simulink model "MCVT_Integration"  (R2020a)
settings: Solver=ode8  StartTime=0.0  StopTime=T_end  FixedStep=dt  RelTol=1e-3  EnableMultiTasking=on
values from neighbouring files (symbols this model uses):
  assumed_power_voltage = 1.25e2    (= 125)    [MCVT_Input_File.m]    (also in MCVT_Run_QuickCheck.m)
  battery_power_toggle = 1    [MCVT_Input_File.m]    (also in MCVT_Run_QuickCheck.m)
  battery_trickle_charge_param = 0.1    [MCVT_Input_File.m]    (also in MCVT_Run_QuickCheck.m)
  battery_trickle_leakage_param = 0.01    [MCVT_Input_File.m]    (also in MCVT_Run_QuickCheck.m)
  damage_case_id = 3    [MCVT_Input_File.m]    (also in MCVT_Run_QuickCheck.m = 1)
  dt = 1/fs    (= 0.001)    [MCVT_Run_QuickCheck.m]    (also in MCVT_Sim_Set.m)
  dt_2 = 1/fs_2    (= 0.001)    [MCVT_Run_QuickCheck.m]    (also in MCVT_Sim_Set.m)
  dt_3 = 1/fs_3    (= 0.001)    [MCVT_Run_QuickCheck.m]    (also in MCVT_Sim_Set.m)
  dt_5 = 1/fs_5    (= 0.005)    [MCVT_Run_QuickCheck.m]    (also in MCVT_Sim_Set.m)
  dt_8 = 1/fs_8    (= 0.05)    [MCVT_Run_QuickCheck.m]    (also in MCVT_Sim_Set.m)
  dt_9 = 1/fs_9    (= 0.001)    [MCVT_Run_QuickCheck.m]    (also in MCVT_Sim_Set.m)
  dust_met_duration = 3    [MCVT_Input_File.m]    (also in MCVT_Run_QuickCheck.m = 2)
  ECLSS_indoor_set_temperature = 298.15    [MCVT_Input_File.m]    (also in MCVT_Run_QuickCheck.m)
  energy_store_max_o = 150    [MCVT_Input_File.m]    (also in MCVT_Run_QuickCheck.m)
  energy_store_o = 120    [MCVT_Input_File.m]    (also in MCVT_Run_QuickCheck.m)
  fs = 1000    [MCVT_Run_QuickCheck.m]    (also in MCVT_Sim_Set.m)
  gain_charge_param = 1    [MCVT_Input_File.m]    (also in MCVT_Run_QuickCheck.m)
  ground_temperature = 373    [MCVT_Habitat_Design_Input_File.m]    (also in MCVT_Run_QuickCheck.m)
  I_max_charge = 800    [MCVT_Input_File.m]    (also in MCVT_Run_QuickCheck.m)
  I_max_discharge = 800    [MCVT_Input_File.m]    (also in MCVT_Run_QuickCheck.m)
  initial_pressure = 1e5    (= 100000)    [MCVT_Input_File.m]    (also in MCVT_Run_QuickCheck.m)
  initial_temperature = 295    [MCVT_Input_File.m]    (also in MCVT_Run_QuickCheck.m)
  interior_leak_rate = 0.00415    [MCVT_Input_File.m]    (also in MCVT_Run_QuickCheck.m)
  launch_dust_accum_start_time = 10    [MCVT_Input_File.m]    (also in MCVT_Run_QuickCheck.m = 2)
  launch_dust_accum_stop_time = 12    [MCVT_Input_File.m]    (also in MCVT_Run_QuickCheck.m = 3)
  meteorite_impact_time = 2    [MCVT_Input_File.m]    (also in MCVT_Run_QuickCheck.m = 1)
  nuclear_power_toggle = 1    [MCVT_Input_File.m]    (also in MCVT_Run_QuickCheck.m)
  print_interval = 30    [MCVT_Input_File.m]
  SOC_lower_thres = 0.5    [MCVT_Input_File.m]    (also in MCVT_Run_QuickCheck.m)
  SOC_upper_thres = 0.9    [MCVT_Input_File.m]    (also in MCVT_Run_QuickCheck.m)
  solar_power_toggle = 1    [MCVT_Input_File.m]    (also in MCVT_Run_QuickCheck.m)
  space_temperature = 4    [MCVT_Habitat_Design_Input_File.m]    (also in MCVT_Run_QuickCheck.m)
  Start_angle = 90    [MCVT_Input_File.m]    (also in MCVT_Run_QuickCheck.m)
undefined symbols (not defined in the file or in its folder): A_flow_restrictor, ACTIVITY_LOOKUP_TABLE, air, Aref, Bmap, Bmap2, Bmap3, comms, DiscIntNeverNeededParam, DmgSPL, dust_parameters, ECLSS_dust_damage_repair_time, ECLSS_heater_capacity, ECLSS_IPS_restrictor, ECLSS_IPS_setpointPressure, ECLSS_paint_damage_repair_time, ECLSS_radiatorLoop_discreteNumber, ECLSS_Vst, emptyActivity, expected_heat_add, FI, habitat, HIEM2SMM, HIEM2STM, HIEMDamageElements, impact_numbering, initial_SPLT_damage_index, input_air, input_Cond, input_CP, input_discretization, input_EV, input_Evap, input_radiatorLoop, K_ECLSS_dust_single, K_ECLSS_paint_single, K_Structure_damage_single, Kref, kW_to_W, meteoroidDustDep, mFRF, ndelays, nominalDustDep, noverlap, nuclear_toggle_gain, output_limits, panel_paint0, Ps0, reference_temperature, RepairTime_ECLSS_factor, Sensor_Location_SMM, SMM_T0, SMM_T1, SMM_T2, SMM_T3, SMM_T4, SMM_T4_0, SMM_T4_1, SMM_T4_2, solar_FDD_K ... (+73)
libraries: neural x350, simulink x39, xpcutilitieslib x7, xpcrtudplib x7, dspstat3 x1
(part 39 of 43)

== matlab function MCVT_Integration/Sys9 - Exterior Environment/Exterior Environment/3D Solar Irradiation model (v9) 1 ==
  data: intime input; xyz_SR output; Sb_tot output; SR_ECLSS output; Sttime input; SR_STM_ei_th2 output; Sb_fin output; Sb_fin_vec output
%Determination of solar irradiation on dome surface, W/m2%

function [xyz_SR,Sb_tot, SR_ECLSS,SR_STM_ei_th2,Sb_fin,Sb_fin_vec] =Solarirradiationver2(intime,Sttime)

    %% Documentation
% Purpose: To determine the Solar Radiation Values at different points of the
%          Structural Protective Layer (SPL)that covers the Structural 
%          Habitat Structure. For more details, please look into the
%          Chapter 2.2 of the documentation report entitled,"Solar Irradiation
%           Model." 
%         
% Date Created: 20 September 2020
% Date Last Modified: 31 July 2021
% Modeler Name: Sachin Tripathi (UConn)
% Funding Acknowledgement: Funded by the NASA RETHi Project

% Version Number: MCVT v4

% Subsystem Connections: 
    %Inputs: No inputs;
    %Outputs: Subsystems 2 (2.2: STM), and 5 (ECLSS);

% Function Dependencies: No Function Dependencies
%   

% Data Dependencies: No Data Dependencies
    
    %Local model specific variables%
    nph=10;  % Number of elements in Φ direction
    nth=6; % Number of elements in Θ direction
    
    %Initializing the values%
    theta_cell=zeros(nph,nth); %Initializing theta_cell%
    cs_thi=zeros(nph,nth); %Initializing cs_thi%
    fx=zeros(nph,nth); %Initializing fx%
    Sb=zeros(nph,nth); 
    Sb_top=0;
    thetas=0;
%     thetas2=0;
%     Sb_top2=0;
    
    
    %Geometric parameters%
    R=2.5; %Inner radius of the dome, m%
    d=0.4; %Thickness of the dome, m%
    d_reg=0.2; %Thickness of the regolith dome, m%
    R_out=R+d; %Outer radius of the dome, m%
    n_d=3; %Division number of the thickness%
    r1=R+d+(n_d-1)*d_reg/n_d;%Radial distance from origin to the base of the surface (regolith) element%
    r2=r1+(d_reg/n_d)/2; %Radial distance from origin to the center of the surface (regolith) element%
     
%Climatic Conditions%
    del=0; %Angle of declination of sun, degrees%
    lam=0; %Local latitude due north, degrees (°)%
%     abs_0=0.6; %Absorptance of concrete at thetai=0%
%     abs_c0=0.6; %Absorptance of concrete at thetai=0%
    Gs_max=1450; %Maximum solar intensity, W/m^2%
    r_reg=0.13; %Reflectance of lunar regolith is 13%%

    %Determination of the position of each of the cell%
    dphi=360/nph;    %Circumferential angle of one differential element, degrees(°)%
    dtheta=90/nth;   %Differential angle in the theta direction, degrees(°)%

    
   dtime=6720; %1 degree of sun rotation in mooon is equal to 6720 seconds%
   s_inc=(intime+Sttime)/6720; %Converting intime in seconds into degrees%
%    thetac=2; %theta c in degrees%
   
   
   st_phi=dphi/2; %Starting point of phi%
   st_theta=dtheta/2;%Starting point of theta%

%Determination of the coordinates of the each cells%
  phi=st_phi:dphi:360-st_phi; %Creating a vector of phi, central point, , degrees(°)%
  theta=st_theta:dtheta:90-dtheta/2; %Creating a vector of theta, central point, degrees(°)%

    theta_cell(:,:)=repmat(theta,length(phi),1); %Creating the matrix of theta of cells, degrees(°)%
    
    phi_cell=repmat(phi',1,length(theta)); %Creating a matrix of phi of cell, degrees(°)%
    tot_cells=length(theta)*length(phi); %Total number of cells%

    %% Creating a normal vector of all cells
    nd1=cosd(theta_cell); %First component of normal vector%
    nd2=-sind(theta_cell).*sind(phi_cell); %Second component of normal vector%
    nd3=-sind(theta_cell).*cosd(phi_cell); %Third component of normal vector%

    
  %% Determination of the Sun's position vector  
    omega=s_inc-90; %Hour angle, degrees(°)%

    %Determination of zenith angle%
    cs_thz=cosd(del)*cosd(lam)*cosd(omega)+sind(del)*sind(lam); %Determination of zenith angle, degrees(°)%

    ns1=cs_thz; %First component of sun's position vector%
    ns2=-cosd(del)*sind(omega); %Second component of sun's position vector%
    ns3=-cosd(del)*cosd(omega)*sind(lam)+sind(del)*cosd(lam); %Third component of sun's position vector%

    %Determination of the angle of incidence, thetai, for all elements at different position of sun%
    %cs_thi gives the cos (thi), where 'thi' is the angle between the sun's position vector and the dome normal vector%
    
    cs_thi =nd1.*ns1+nd2.*ns2+nd3.*ns3; %Creating cos thetai which is the angle between the sun's position vector and the dome nomal vectors, cos (degrees(°)) %

    thi=acosd(cs_thi); %Geting angle between sun's position vector and the normal to the differential elements, degrees(°) by inversing cos_thi() function%

%     %Determination of absorptance%
%     ra_abs=1-1.5879e-3*thi+2.7314e-4*thi.^2-2.2026e-5*thi.^3+9.0244e-7*thi.^4-1.8e-8*thi.^5+1.7734e-10*thi.^6-6.9937e-13*thi.^7;
%     abs_thi=ra_abs.*abs_0; %Resulting absorptance value%
    
    %% Creating a control function%
    %Control function, fx, takes into account of the shadow effect i.e. if
    %cs_thi is negative, the element will be under shadow and viceversa
        fx=(cs_thi>0); %This produces the logical array with the 0's and 1's; when it is true, returns 1 and 0, for false returns%
        fx=double(fx); %COnverting the logical array to the double precision/ data type%
        
    %Final calculation of the solar irradiation, Sb, W/m2 discarding the diffuse component%
    Sb= fx.*Gs_max.*cs_thi.*1; %Direct solar beam radiation without considering absorptance, W/(m^2)% 
 
      
    %For element at top of the dome, (for ECLSS system) as well as  
    %Assume lunar regolith as the flat surface%
    %Determination of solar angle of incidence% 
    if s_inc<=90 %if the solar angle of incidence is <=90°%
        thetas=90-s_inc;
        
        elseif s_inc>90 %if the solar angle of incidence is >90°%
         thetas=s_inc-90;

    end
    
%     %Determination of absorptance for the top element only%
%     ra_abstop=1.-1.5879e-3.*thetas+2.7314e-4.*thetas.^2-2.2026e-5.*thetas.^3+9.0244e-7.*thetas.^4-1.8e-8.*thetas.^5+1.7734e-10.*thetas.^6-6.9937e-13.*thetas.^7;
%     abs_thi_top=ra_abstop.*abs_c0; %Resulting absorptance value%
    
    
    Sb_top=Gs_max.*1.*cosd(thetas); %Calculation of the solar radiation of the dome top elements, W/(m^2)%
    
    
    %%%%%%%%%%%%%%%%%%%%%%%%%%%%%%%%%%%%%%%%%%%%%%%%%%%%%%%%%%%%%%%%%%%%%%%%
    %% Determination of the solar albedo from the lunar regolith%
   
    %Determination of solar albedo reflection from lunar regolith%
   
    q_lun_albed_reg=r_reg*Gs_max*cosd(thetas); %Determination of the solar albedo reflection, W/m^2%

    %Since the direction of the solar albedo reflected from ground is unknown, this amount is added as such for all differential elements%
    %End of the determination of the solar albedo calculations%
    %*************************************************************************%
    %Getting total solar irradiation on the element with sum of direct beam radiation and the solar albedo%
   
     S_tot_in=Sb+q_lun_albed_reg; %Total sum of direct solar and albedo%

   

            
    
    %%%%%%%%%%%%%%%%%%%%%%%%%%%%%%%%%%%%%%%%%%%%%%%%%%%%%%%%%%%%%%%%%%%%%%

%******Data arrangement*********%
theta_mat=reshape(theta_cell,[],1); %Converting theta_matrix to column vector%
phi_mat=reshape(phi_cell,[],1);     %Converting phi_matrix to column vector%
rad_mat= r2.*ones(tot_cells,1);     %Converting rad_matrix to column vector, outer cell%
position_info=[rad_mat theta_mat phi_mat ]; %Creating 99by 3 matrix in which each column are radius, theta and phi value%
Sb_tot=[Sb_top;reshape(Sb',[],1)];

%Conversion into the universal cartesian coordinates%
%Step 1: Conversion into cartesian coordinates for the given parent coordinate i.e. SOuth=+X, and West=+Y%
x_par=rad_mat.*sind(theta_mat).*cosd(phi_mat); %X-coordinate, parent axes, m%
y_par=rad_mat.*sind(theta_mat).*sind(phi_mat); %Y-coordinate, parent axes, m%
z_par=rad_mat.*cosd(theta_mat); %Z-coordinate, parent axes, m%
xyz_par=[x_par y_par z_par]; %Matrix of cartesian coordinates based upon the parent axes, size (:,3)%

% %Step 2: Conversion of cartesian coordinates from the given parent
% %axes to the universal axes as SMM model%
%Step 1: Alter X and Y-coordinates in the parent coordinates%
xyz_ch=[-y_par z_par x_par]; %Adjusting xyz_coordinates%

xyz_SR=xyz_ch; %Cartesian coordinates, size (:,3), in the vector form%
% xyz_SR=[[0 3.067 0]; xyz_SR];

% 
% RMx=[1 0 0; 0 cos(+90/180*pi) -sin(+90/180*pi); 0 sin(+90/180*pi) cos(+90/180*pi)]; % rotation matrix
% xyz_SR=zeros(size(xyz_SR_unrot,1),size(xyz_SR_unrot,2));
% for i=1:size(xyz_SR_unrot,1)
%     xyz_SR(i,:)=xyz_SR_unrot(i,:)*RMx; % Rotation matrix w.r.t x-axis by Seungwook
% end

SR_ECLSS=Sb_top;

% %% For ECLSS fake made increased by 60 degrees, 05042021%
% %For top element%
%     if s_inc2<=90
%         thetas2=90-s_inc2;
%         
%         elseif s_inc2>90
%          thetas2=s_inc2-90;
% 
%     end
% 
% Sb_top2=Gs_max.*1.*cosd(thetas2); %Fake Solar Radiation at top%
% SR_ECLSSfake=Sb_top2;
    
SR_STM_ei_th2=S_tot_in(8,3);
Sb_fin=S_tot_in;
Sb_fin_vec=reshape(Sb_fin,[],1); %Converting the matrix form to the vector form%

end

== matlab function MCVT_Integration/Sys2 - Structural System/Sys2_Structural_System/printRepair ==
  data: x input
function printRepair(x)


if x(1)>0
    disp('        Structural System is Repaired');
end   


end

== matlab function MCVT_Integration/Sys2 - Structural System/Sys2_Structural_System/Fault Detection and Diagnosis (FDD) 2.3/Accelerometer/Accelerometer/Damage State Control Block ==
  data: state_number input; y output; Acc input; Time input
function y = fcn(state_number,Acc,Time)

for i = 1:size(state_number,1)
    if state_number(i) == 1
        Acc(i*3-2:i*3) = Acc(i*3-2:i*3);
    else
        if state_number(i) == 2
            temp = Acc(i*3-2:i*3) - 12;
            Acc(i*3-2:i*3) = temp * 0.1 + 12;
        else
            if state_number(i) == 3
                if Time>=11 && Time<=14
                    Acc(i*3-2:i*3) = NaN;
                end
            else
                if state_number(i) == 4
                    Acc(i*3-2:i*3) = NaN;
                end
            end
        end
    end
end

y = Acc;

== matlab function MCVT_Integration/Sys2 - Structural System/Sys2_Structural_System/Fault Detection and Diagnosis (FDD) 2.3/Accelerometer/Accelerometer/Switch ON//OFF Control Block/Switch OFF/MATLAB Function1 ==
  data: sensor input; u input; y output; Time input
function y = fcn(sensor,u,Time)

for i = 1:size(sensor,1)
    if Time>=0
        u(sensor(i)*3-2:sensor(i)*3) = NaN;
    end
end

y = u;

== matlab function MCVT_Integration/Sys2 - Structural System/Sys2_Structural_System/Fault Detection and Diagnosis (FDD) 2.3/Accelerometer Power Consumption ==
  data: p input; y output
function y = fcn(p)

if p >= 1.2*10^(-4)*3
    u = 1.2*10^(-4)*3;
else
    u = 0;
end

y = u;

== matlab function MCVT_Integration/Sys2 - Structural System/Sys2_Structural_System/Fault Detection and Diagnosis (FDD) 2.3/Data Anomaly Detection/Data Anomaly Detection ==
  data: Acc input; indicator output; max_value output; num_nan output; y output
function [indicator,max_value,num_nan,y] = fcn(Acc)

indicator = zeros(9,1);
num_nan = zeros(9,1);
max_value = zeros(9,1);

for i = 1:9
    
    y = Acc(:,i);
    TF = isnan(Acc(:,i));
    num_nan(i) = sum(TF);
    max_value(i) = max(abs(Acc(:,i)));
    
    if num_nan(i) == 0
        indicator(i) = 1;
    else
        if num_nan(i) >= 1 && num_nan(i) < 5120
            indicator(i) = 3;
        else
            if num_nan(i) == 5120
                indicator(i) = 4;
            end
        end
    end
    
    if indicator(i) == 1 && max_value(i) <= 3e-3 || max_value(i) >= 0.05
        indicator(i) = 2;
    end   
end

== matlab function MCVT_Integration/Sys2 - Structural System/Sys2_Structural_System/Fault Detection and Diagnosis (FDD) 2.3/FRF from Accelerometer 1/FRF Calculation ==
  data: exc input; frf output; acc input; m input; noverlap input; w input
function frf = xfunc(exc,acc,m,noverlap,w)

%  [XFER21,FREQV,P] = XFUNC(X,Y,FS,M,NOVERLAP) will estimate the
%	complex transfer function given time histories of the
%	input, X, and the output, Y. Given the complex response,
%	the magnitude and phase can be determined by using the
%	ABS and ANGLE functions. FS is the sampling frequency (Hz)
%	at which the data was taken. The X and Y sequences of
%	N points are divided into NS sections of M points each
%	(M must be a power of two). Using an M-point FFT,
%	successive sections are Hanning windowed, FFT'd and
%	averaged.	** SOME PARTS FROM SPECTRUM FUNCTION
%
%	XFUNC(X,Y,FS) 	--> NO AVERAGING
%	XFUNC(X,Y,FS,M)	--> AVERAGING BUT NO OVERLAP
%	NO OUTPUT ARGUMENTS WILL CREATE A BODE PLOT
%
% 	The output P is the matrix of power spectrums in the order
%	P = [Pxx,Pyy,Pxy].
%
%	NOTES: 	Xfer data may be written to a specified file.
%		Variables were chosen to immitate certain IP names.
%		Depending on the anti-aliasing filter used a certain
%			percentage of the data will include aliased
%			data (IP is 78% correct).

% COMMENTS
% nargin = 3 --> no overlap, no averaging
% nargin = 4 --> no overlap, averaging data
% nargin = 5 --> some overlap, and averaging data
% set color variable to easily change plot color
% may also write data to a file

frf = zeros(1,256*3);
x = exc;

for k = 1:1:3
    y = acc(:,k);
    
%     color	= 'b';
%     
%     if (nargin == 3), m = length(y); noverlap = 0; fmax = fs/2; end
%     if (nargin == 4)
%         if (length(y) == 1)
%             noverlap = m;
%             m = length(y);
%         else
%             noverlap = 0;
%         end
%         fmax = fs/2;
%     end
    %keyboard
    x 	= x(:);		% Make sure x and y are column vectors
    y 	= y(:);
    
    % DEFINE CONSTANTS
     n 	= max(size(x));	% Number of data points in entire time history
     ns 	= fix((n-noverlap)/(m-noverlap));	% Number of samples (frames)
    % (k = fix(n/m) for noverlap=0)
%     w 	= hann(m);	% hanning is replaced by hann
    % Type of windowing: change this for application
    % (Try HAMMING, BLACKMAN, BARTLETT, or your own)
    % HANNING is best for narrow-band random !!
     KMU 	= ns*norm(w)^2;	% Norm. scale factor (AVERAGING & WINDOWING)
     index 	= 1:m;
    % fprintf(1,['\n%5i',' Samples Averaged\n'],ns)
    
    
    % FIND POWER SPECTRUMS OF EACH SAMPLE AND SUM
    Pxx = zeros(m,1)+0i; 
%     Pyy = Pxx; 
    Pxy = Pxx; % Plus 0i
    for i = 1:ns
        xw 	= w.*x(index);		% detrend? no difference
        yw 	= w.*y(index);
        index 	= index + (m - noverlap);
        Xx 	= fft(xw);
        Yy 	= fft(yw);
        Pxx 	= Pxx + Xx.*conj(Xx);
%         Pyy 	= Pyy + Yy.*conj(Yy);
        Pxy 	= Pxy + Yy.*conj(Xx);
    end
    
    % SELECT
    %select = [1:m/2];
    select = [1:m/2+1];   % include DC AND Nyquist
    %FreqV	= [fs/2/(m/2):fs/2/(m/2):fs/2]';		% (Nyquist)
%     FreqV	= (select - 1)'*fs/m;
%     mcorrect	= round(.78*max(select));		% first 78%
%     fcorrect	= FreqV(mcorrect);
    
    % AVERAGED & SCALED DATA
    Pxx = Pxx(select)./KMU;			% Double sided
%     Pyy = Pyy(select)./KMU;
    Pxy = Pxy(select)./KMU;
%     P   = [Pxx,Pyy,Pxy];
    
    Xfer21 	= Pxy./Pxx;
    frf(1,256*(k-1)+1:256*k) = abs(Xfer21(1:256,1));
end

% %keyboard
% % NO OUTPUT ARGUMENTS, PLOT BODE
% if (nargout == 0),
%     
%     fig1	= gcf;
%     subplot(211)
%     plot(FreqV,db(Xfer21),color);grid
%     xlabel('Frequency (Hz)')
%     ylabel('Gain (dB)')
%     title('Transfer Function')
%     limits1	= axis;hold on
%     plot([fcorrect,fcorrect],[limits1(3),limits1(4)],'r');
%     xlim([0,fmax]);
%     
%     hold off
%     
%     subplot(212)
%     plot(FreqV,angle(Xfer21)*180/pi,color);grid
%     xlabel('Frequency (Hz)')
%     ylabel('Phase (deg)')
%     limits2	= axis;hold on
%     plot([fcorrect,fcorrect],[limits2(3),limits2(4)],'r');
%     hold off
%     axis([0 fmax -200 50]);
%     %	orient tall;print
%     return % Suppress output
% end

== matlab function MCVT_Integration/Sys2 - Structural System/Sys2_Structural_System/Fault Detection and Diagnosis (FDD) 2.3/FRF from Accelerometer 2/FRF Calculation ==
  data: exc input; frf output; acc input; m input; noverlap input; w input
function frf = xfunc(exc,acc,m,noverlap,w)

%  [XFER21,FREQV,P] = XFUNC(X,Y,FS,M,NOVERLAP) will estimate the
%	complex transfer function given time histories of the
%	input, X, and the output, Y. Given the complex response,
%	the magnitude and phase can be determined by using the
%	ABS and ANGLE functions. FS is the sampling frequency (Hz)
%	at which the data was taken. The X and Y sequences of
%	N points are divided into NS sections of M points each
%	(M must be a power of two). Using an M-point FFT,
%	successive sections are Hanning windowed, FFT'd and
%	averaged.	** SOME PARTS FROM SPECTRUM FUNCTION
%
%	XFUNC(X,Y,FS) 	--> NO AVERAGING
%	XFUNC(X,Y,FS,M)	--> AVERAGING BUT NO OVERLAP
%	NO OUTPUT ARGUMENTS WILL CREATE A BODE PLOT
%
% 	The output P is the matrix of power spectrums in the order
%	P = [Pxx,Pyy,Pxy].
%
%	NOTES: 	Xfer data may be written to a specified file.
%		Variables were chosen to immitate certain IP names.
%		Depending on the anti-aliasing filter used a certain
%			percentage of the data will include aliased
%			data (IP is 78% correct).

% COMMENTS
% nargin = 3 --> no overlap, no averaging
% nargin = 4 --> no overlap, averaging data
% nargin = 5 --> some overlap, and averaging data
% set color variable to easily change plot color
% may also write data to a file

frf = zeros(1,256*3);
x = exc;

for k = 1:1:3
    t = k+3;
    y = acc(:,t);
    
%     color	= 'b';
%     
%     if (nargin == 3), m = length(y); noverlap = 0; fmax = fs/2; end
%     if (nargin == 4)
%         if (length(y) == 1)
%             noverlap = m;
%             m = length(y);
%         else
%             noverlap = 0;
%         end
%         fmax = fs/2;
%     end
    %keyboard
    x 	= x(:);		% Make sure x and y are column vectors
    y 	= y(:);
    
    % DEFINE CONSTANTS
     n 	= max(size(x));	% Number of data points in entire time history
     ns 	= fix((n-noverlap)/(m-noverlap));	% Number of samples (frames)
    % (k = fix(n/m) for noverlap=0)
%     w 	= hann(m);	% hanning is replaced by hann
    % Type of windowing: change this for application
    % (Try HAMMING, BLACKMAN, BARTLETT, or your own)
    % HANNING is best for narrow-band random !!
     KMU 	= ns*norm(w)^2;	% Norm. scale factor (AVERAGING & WINDOWING)
     index 	= 1:m;
    % fprintf(1,['\n%5i',' Samples Averaged\n'],ns)
    
    
    % FIND POWER SPECTRUMS OF EACH SAMPLE AND SUM
    Pxx = zeros(m,1)+0i; 
%     Pyy = Pxx; 
    Pxy = Pxx; % Plus 0i
    for i = 1:ns
        xw 	= w.*x(index);		% detrend? no difference
        yw 	= w.*y(index);
        index 	= index + (m - noverlap);
        Xx 	= fft(xw);
        Yy 	= fft(yw);
        Pxx 	= Pxx + Xx.*conj(Xx);
%         Pyy 	= Pyy + Yy.*conj(Yy);
        Pxy 	= Pxy + Yy.*conj(Xx);
    end
    
    % SELECT
    %select = [1:m/2];
    select = [1:m/2+1];   % include DC AND Nyquist
    %FreqV	= [fs/2/(m/2):fs/2/(m/2):fs/2]';		% (Nyquist)
%     FreqV	= (select - 1)'*fs/m;
%     mcorrect	= round(.78*max(select));		% first 78%
%     fcorrect	= FreqV(mcorrect);
    
    % AVERAGED & SCALED DATA
    Pxx = Pxx(select)./KMU;			% Double sided
%     Pyy = Pyy(select)./KMU;
    Pxy = Pxy(select)./KMU;
%     P   = [Pxx,Pyy,Pxy];
    
    Xfer21 	= Pxy./Pxx;
    frf(1,256*(k-1)+1:256*k) = abs(Xfer21(1:256,1));
end

% %keyboard
% % NO OUTPUT ARGUMENTS, PLOT BODE
% if (nargout == 0),
%     
%     fig1	= gcf;
%     subplot(211)
%     plot(FreqV,db(Xfer21),color);grid
%     xlabel('Frequency (Hz)')
%     ylabel('Gain (dB)')
%     title('Transfer Function')
%     limits1	= axis;hold on
%     plot([fcorrect,fcorrect],[limits1(3),limits1(4)],'r');
%     xlim([0,fmax]);
%     
%     hold off
%     
%     subplot(212)
%     plot(FreqV,angle(Xfer21)*180/pi,color);grid
%     xlabel('Frequency (Hz)')
%     ylabel('Phase (deg)')
%     limits2	= axis;hold on
%     plot([fcorrect,fcorrect],[limits2(3),limits2(4)],'r');
%     hold off
%     axis([0 fmax -200 50]);
%     %	orient tall;print
%     return % Suppress output
% end

== matlab function MCVT_Integration/Sys2 - Structural System/Sys2_Structural_System/Fault Detection and Diagnosis (FDD) 2.3/FRF from Accelerometer 3/FRF Calculation ==
  data: exc input; frf output; acc input; m input; noverlap input; w input
function frf = xfunc(exc,acc,m,noverlap,w)

%  [XFER21,FREQV,P] = XFUNC(X,Y,FS,M,NOVERLAP) will estimate the
%	complex transfer function given time histories of the
%	input, X, and the output, Y. Given the complex response,
%	the magnitude and phase can be determined by using the
%	ABS and ANGLE functions. FS is the sampling frequency (Hz)
%	at which the data was taken. The X and Y sequences of
%	N points are divided into NS sections of M points each
%	(M must be a power of two). Using an M-point FFT,
%	successive sections are Hanning windowed, FFT'd and
%	averaged.	** SOME PARTS FROM SPECTRUM FUNCTION
%
%	XFUNC(X,Y,FS) 	--> NO AVERAGING
%	XFUNC(X,Y,FS,M)	--> AVERAGING BUT NO OVERLAP
%	NO OUTPUT ARGUMENTS WILL CREATE A BODE PLOT
%
% 	The output P is the matrix of power spectrums in the order
%	P = [Pxx,Pyy,Pxy].
%
%	NOTES: 	Xfer data may be written to a specified file.
%		Variables were chosen to immitate certain IP names.
%		Depending on the anti-aliasing filter used a certain
%			percentage of the data will include aliased
%			data (IP is 78% correct).

% COMMENTS
% nargin = 3 --> no overlap, no averaging
% nargin = 4 --> no overlap, averaging data
% nargin = 5 --> some overlap, and averaging data
% set color variable to easily change plot color
% may also write data to a file

frf = zeros(1,256*3);
x = exc;

for k = 1:1:3
    t = k+6;
    y = acc(:,t);
    
%     color	= 'b';
%     
%     if (nargin == 3), m = length(y); noverlap = 0; fmax = fs/2; end
%     if (nargin == 4)
%         if (length(y) == 1)
%             noverlap = m;
%             m = length(y);
%         else
%             noverlap = 0;
%         end
%         fmax = fs/2;
%     end
    %keyboard
    x 	= x(:);		% Make sure x and y are column vectors
    y 	= y(:);
    
    % DEFINE CONSTANTS
     n 	= max(size(x));	% Number of data points in entire time history
     ns 	= fix((n-noverlap)/(m-noverlap));	% Number of samples (frames)
    % (k = fix(n/m) for noverlap=0)
%     w 	= hann(m);	% hanning is replaced by hann
    % Type of windowing: change this for application
    % (Try HAMMING, BLACKMAN, BARTLETT, or your own)
    % HANNING is best for narrow-band random !!
     KMU 	= ns*norm(w)^2;	% Norm. scale factor (AVERAGING & WINDOWING)
     index 	= 1:m;
    % fprintf(1,['\n%5i',' Samples Averaged\n'],ns)
    
    
    % FIND POWER SPECTRUMS OF EACH SAMPLE AND SUM
    Pxx = zeros(m,1)+0i; 
%     Pyy = Pxx; 
    Pxy = Pxx; % Plus 0i
    for i = 1:ns
        xw 	= w.*x(index);		% detrend? no difference
        yw 	= w.*y(index);
        index 	= index + (m - noverlap);
        Xx 	= fft(xw);
        Yy 	= fft(yw);
        Pxx 	= Pxx + Xx.*conj(Xx);
%         Pyy 	= Pyy + Yy.*conj(Yy);
        Pxy 	= Pxy + Yy.*conj(Xx);
    end
    
    % SELECT
    %select = [1:m/2];
    select = [1:m/2+1];   % include DC AND Nyquist
    %FreqV	= [fs/2/(m/2):fs/2/(m/2):fs/2]';		% (Nyquist)
%     FreqV	= (select - 1)'*fs/m;
%     mcorrect	= round(.78*max(select));		% first 78%
%     fcorrect	= FreqV(mcorrect);
    
    % AVERAGED & SCALED DATA
    Pxx = Pxx(select)./KMU;			% Double sided
%     Pyy = Pyy(select)./KMU;
    Pxy = Pxy(select)./KMU;
%     P   = [Pxx,Pyy,Pxy];
    
    Xfer21 	= Pxy./Pxx;
    frf(1,256*(k-1)+1:256*k) = abs(Xfer21(1:256,1));
end

% %keyboard
% % NO OUTPUT ARGUMENTS, PLOT BODE
% if (nargout == 0),
%     
%     fig1	= gcf;
%     subplot(211)
%     plot(FreqV,db(Xfer21),color);grid
%     xlabel('Frequency (Hz)')
%     ylabel('Gain (dB)')
%     title('Transfer Function')
%     limits1	= axis;hold on
%     plot([fcorrect,fcorrect],[limits1(3),limits1(4)],'r');
%     xlim([0,fmax]);
%     
%     hold off
%     
%     subplot(212)
%     plot(FreqV,angle(Xfer21)*180/pi,color);grid
%     xlabel('Frequency (Hz)')
%     ylabel('Phase (deg)')
%     limits2	= axis;hold on
%     plot([fcorrect,fcorrect],[limits2(3),limits2(4)],'r');
%     hold off
%     axis([0 fmax -200 50]);
%     %	orient tall;print
%     return % Suppress output
% end

== matlab function MCVT_Integration/Sys2 - Structural System/Sys2_Structural_System/Impact_Force_&_SPL_Damage ==
  data: Fimp output; FI_index input; dmg_case input; repair_status input; DmgHist input; FI parameter; Dmg output; DmgSPL parameter; DmgHist output
function [Fimp,Dmg,DmgHist]   = Impact_Force_and_SPL_Damage(FI_index,dmg_case,repair_status,DmgHist, FI, DmgSPL)

% NOTE: impact force-time profile is given for the sampling time of 0.001 sec

Fimp=0;
Dmg=0;

if FI_index(1,1) == 1
    
    Fimp=FI(FI_index(1,2),dmg_case);
    Dmg=DmgSPL(FI_index(1,2),dmg_case);
    DmgHist=Dmg;
    
end

if repair_status == 0
    Dmg=DmgHist;
else
    Dmg=0;
end



end

== matlab function MCVT_Integration/Sys2 - Structural System/Sys2_Structural_System/FDD adjustment/FDDS ==
  data: Y output; x input; t input
function Y = FDDS(x, t)

if t<6
    Y=6;
else
    [M,I] = max(x);
    Y=I;
end

end

== matlab function MCVT_Integration/Sys3 - Power System/Power Generation Subsystem1/Solar Power Generation/MATLAB Function ==
  data: Dratio input; DustAccu output
function DustAccu = fcn(Dratio)
% DustAccu=0 : health
% DustAccu=1 : minor
% DustAccu=2 : medium
% DustAccu=3 : major

if 0<=Dratio && Dratio<0.10
    DustAccu=0;
elseif 0.10<=Dratio && Dratio<0.30
    DustAccu=1;
elseif 0.30<=Dratio && Dratio<0.70
    DustAccu=2;
else
    DustAccu=3;
end

== matlab function MCVT_Integration/Sys2 - Structural System/Sys2_Structural_System/FDD adjustment/FDDSr ==
  data: Y output; x input
function Y = adjust(x)

 Y=mode(x);
 
 if sum(x(:) == Y)<8000
     Y=min(x);
 end
 
 if Y==0
     Y=6;
 end
 

 
end

== matlab function MCVT_Integration/Sys8 - Interior Environment/HIEM_Damage_Indication ==
  data: DamageInfo input; Damage output
function Damage  = HIEM_Damage_Indication(DamageInfo)

Damage=sign(DamageInfo);

end

== matlab function MCVT_Integration/Sys9 - Exterior Environment/Exterior Environment/Dust/DustDep ==
  data: exhaustEvent input; meteoroidEvent input; nominalDustDep input; meteoroidDustDep input; dist input; tao_total output; input_L input; countp1 input; count1 output; countp2 input; count2 output
function [tao_total,count1, count2]=dust_deposition_event(exhaustEvent, meteoroidEvent, nominalDustDep, meteoroidDustDep, dist, input_L, countp1, countp2)
    %{
        #=================================================#
        #    Code developed for the RETH-Institute        #
        #    By: Chunjian Pan                             #
        #    Post Doctoral Researcher @ Purdue University #
        #                                                 #
        #    Code Extended:                               #
        #    By: Murali Krishnan R   & Yuguang Fu         #
        #    PhD Student @ Purdue University              #
        #=================================================#
    
    Input
    -----
    :event: Indicator function for the exhaust event
    :d: Distance away from a landing or launch site, unit:m
    :input_L: List of Parameter Values for the Model

    %% output
    % tao_L:   Dust deposition at a radius `d` meters from launch event, unit: mg/cm^2
    
    %}
    

    %% Extract parameter values from input list

    g=input_L(1);                   % Gravitational acceleration
    v0_L=input_L(2);                % Initial dust particle speed
    C_L=input_L(3);                 % Dust intensity parameter for engine exhaust, unit: mg/cm^2/s-engine exhaust 
    beta_L=input_L(4);              % Parameter to control a dust particle's flying trajectory

    nC = size(dist,1); % number of locations of interest
    tao_L = zeros(nC, 1);
    tao_M = zeros(nC, 1);
    %%
    
    count1=0;
    count2=0;
    
    if exhaustEvent
        count1=countp1+1;
        if count1==1
            disp('        Dust Accumulation Starts due to Engine Exhaustive Events');
        end
        
        for i=1:nC
            AA=C_L*(dist(i)*g).^0.5/v0_L;
            BB=exp(-beta_L.*(dist(i)*g).^0.5/v0_L);
            tao_L(i)=AA.*BB;
        end
    end
    
    if meteoroidEvent
        count2=countp2+1;
        if count2==1
            disp('        Dust Accumulation Starts due to Meteorite Impacts');
        end
        tao_M=meteoroidDustDep;
    end
    
    tao_total = tao_L + tao_M + nominalDustDep;
end

== stateflow chart MCVT_Integration/Sys3 - Power System/Power Generation Subsystem1/Nuclear Power Generation/Disturbances1/Chart1 ==
  state update_random_input
    en,du: [ sec_flux, row, prim_flow, sec_flow] =output_exp();
    exit:
  state wait_random_time
    entry: rand_wait_time = randi([2.5,20]);
    during:
    exit:
  (default) -> update_random_input
  update_random_input -> wait_random_time : after(1,tick)
  wait_random_time -> update_random_input : after(rand_wait_time, sec)
  data: sec_loop_fluct function_output; row_gain function_output; prim_flowrate function_output; sec_flowrate function_output; row output =row_gain0; prim_flow output =prim_flowrate0; sec_flow output =sec_flowrate0; sec_flux output =sec_loop_fluct0; rand_wait_time local
  function [sec_loop_fluct, row_gain, prim_flowrate, sec_flowrate]  = output_exp:
    | function [sec_loop_fluct, row_gain, prim_flowrate, sec_flowrate]  = output_exp
    | % 'sec_loop_pct_fluctuation','radioactivity_gain',...
    | %      'primary_loop_flowrate_gain','sec_loop_flowrate_gain','dust disturbance'
    | scale = @(data, l, u, inmin, inmax) l + ((data-inmin)./(inmax-inmin)).*(u-l);
    | 
    | %Disturbances
    | sec_loop_fluct = scale(rand,-0.01,0.01,0,1);
    | row_gain = 0;%scale(rand,-0.001,0.001,0,1);
    | 
    | %Faults
    | prim_flowrate = 1;
    | sec_flowrate = 1; %rand;
    | dust_dist = 1; %
    | 
    | end

== matlab function MCVT_Integration/Sys3 - Power System/Power Generation Subsystem1/Nuclear Power Generation/SP-100 Power Generation/	Power Generation/Interpolation/MATLAB Function ==
  data: dust_lower input; dust_low output; dust_upper input; dust_up output
function [dust_low,dust_up] = fcn(dust_lower,dust_upper)
all_levels = 0:5:100;
if dust_lower <= 0 || dust_upper <= 0
    dust_low = all_levels(1);
    dust_up = all_levels(2);
else
    dust_low = all_levels(dust_lower)/100;
    dust_up = all_levels(dust_upper)/100;
end
end

== matlab function MCVT_Integration/Sys3 - Power System/Power Generation Subsystem1/Nuclear Power Generation/SP-100 Power Generation/	Power Generation/Interpolation/Secondary Loop + Stirling Loop interp/MATLAB Function1 ==
  data: u input; Tc2_lower output
function Tc2_lower = fcn(u)
Tc2_lower = u(:,4);
end

== matlab function MCVT_Integration/Sys3 - Power System/Power Generation Subsystem1/Nuclear Power Generation/SP-100 Power Generation/	Power Generation/Interpolation/Secondary Loop + Stirling Loop lower/MATLAB Function1 ==
  data: u input; Tc2_lower output
function Tc2_lower = fcn(u)
Tc2_lower = u(:,4);
end

== matlab function MCVT_Integration/Sys3 - Power System/Power Generation Subsystem1/Nuclear Power Generation/SP-100 Power Generation/	Power Generation/Interpolation/Secondary Loop + Stirling Loop upper/MATLAB Function1 ==
  data: u input; Tc2_lower output
function Tc2_lower = fcn(u)
Tc2_lower = u(:,4);
end

== matlab function MCVT_Integration/Sys3 - Power System/Power Generation Subsystem1/Nuclear Power Generation/SP-100 Power Generation/	Power Generation/Nominal Power/Secondary Loop + Stirling Loop/MATLAB Function1 ==
  data: u input; Tc2_lower output
function Tc2_lower = fcn(u)
Tc2_lower = u(:,4);
end

== matlab function MCVT_Integration/Sys3 - Power System/Power Generation Subsystem1/Nuclear Power Generation/SP-100 Power Generation/	Power Generation/Nominal Power/Sensor Models/Cold Junction Temperature Sensing Model/ Resistance Temperature Detector/R vs T ==
  data: R input; T output
function T = fcn(R)

T = 1.76520388802152e-09 * R^4 - 2.98990866289637e-07 * R^3 + 0.000987249006463873 * R^2 ...
    + 2.36178487086887 * R - 245.924688605077;

end

== matlab function MCVT_Integration/Sys3 - Power System/Power Generation Subsystem1/Nuclear Power Generation/SP-100 Power Generation/	Power Generation/Nominal Power/Sensor Models/Cold Junction Temperature Sensing Model/ Resistance Temperature Detector/RTD with Self-heat Error/MATLAB Function ==
  data: I input; R input; U output
function U = fcn(I, R)

U = I * R;     % Operating current = 1mA
               % Voltage output U = I*R

== matlab function MCVT_Integration/Sys5 - ECLSS/Active Thermal Control System (ATCS)/Faulty Panels/Dust impact ==
  data: L_dust input; md input; dust_parameters input; dusty_panels output; panel_paint input size=[2,50]
function dusty_panels= dust_impact(L_dust, md, dust_parameters, panel_paint)
%% Documentation
% Purpose: Combine dust damage and paint damage 
% Date Created: 20 June 2020
% Date Last Modified: 27 July 2021
% Modeler Name: Jaewon Park, CJ Pan (Purdue)
% Funding Acknowledgement: Funded by the NASA RETHi Project (80NSSC19K1076)

% Version Number: MCVT v1.4

% Subsystem Connections: No connection.

% INPUTS %
%   - L_dust: list of panel subsections which are damaged by dust, [1x50],
%   type: integer
%   - md: dust accumulation ratio, unit: g/cm^2, type: float
%   - dust_parameters: material property of dust, [1x3], type: float
%   - panel_paint: list of panel subsections which are damaged by paint
%   degradation, [2x50], type: float
    
% OUTPUTS %
%   - dusty_panels: combined list panel property information, [1x151],
%   type: float

% Function Dependencies: No dependency

% No Data Depenencies
%% Combined damage
% L_dust: Panels covered by lunar dust
% md:  Total amount of dust, g/cm^2;
convert_m2cm = 100;      % convert m to cm


rho_dust=0.7;            % Density of dust layer: unit: g/cm^3;
delta_0=md/rho_dust/convert_m2cm; % Thickness of the layer of the dust unit: unit:m

M1=length(L_dust(L_dust ~= 0));
% Dust parameters  

epsilon_d=dust_parameters(1);          % Emissivity of lunar dust
alpha_d=dust_parameters(2);            % Absorptivity of lunar dust
kd=dust_parameters(3);                 % Conductivity of the dust,unit: W/(m*K)

%
if ~isempty(panel_paint)
    epsilon=panel_paint(1,:);
    alpha=panel_paint(2,:);
else
    epsilon = zeros(1, 50);
    alpha = zeros(1, 50);
end

N=length(epsilon);
delta=zeros(1,N);

if L_dust == 0
    % skip below step and just use the properties reeived by the paint
    % damage block
else
    % Radiator panels that are covered by dust:
    L_dust = L_dust(L_dust ~= 0); % remove zeros
    for k=1:M1
        epsilon(L_dust(k))=epsilon_d;   % emissivity 
        alpha(L_dust(k))=alpha_d;       % absorptivity
        delta(L_dust(k))=delta_0;       % thickness of dust
    end
end


%% Dust deposition along with thermal paint degradation input
dusty_panels=zeros(1,1+3*N);

dusty_panels(1)=kd;

for i=1:N
    dusty_panels(1+i)=delta(i);     % thickness of dust
    dusty_panels(N+1+i)=epsilon(i); % emissivity
    dusty_panels(2*N+1+i)=alpha(i); % absorptivity
end



end

== matlab function MCVT_Integration/Sys3 - Power System/Power Generation Subsystem1/Nuclear Power Generation/SP-100 Power Generation/	Power Generation/Nominal Power/Sensor Models/Cold Junction Temperature Sensing Model/ Resistance Temperature Detector/RTD without Self-heat Error/MATLAB Function ==
  data: I input; I output; R input; U output
function [I,U]= fcn(I,R)

U = I * R;     % Operating current = 1mA
               % Voltage output U = I*R

== matlab function MCVT_Integration/Sys3 - Power System/Power Generation Subsystem1/Nuclear Power Generation/SP-100 Power Generation/	Power Generation/Nominal Power/Sensor Models/Cold Junction Temperature Sensing Model/ Resistance Temperature Detector/Self-heat Calculation/  T vs R  ==
  data: R output; T input
function R = fcn(T)

if T >= 0
    R = 100*(1+3.9083*10^(-3)*T-5.775*10^(-7)*T^2);
else
    R = 100*(1+3.9083*10^(-3)*T-5.775*10^(-7)*T^2-4.183*10^(-12)*(T-100)*T^3);
end

== matlab function MCVT_Integration/Sys3 - Power System/Power Generation Subsystem1/Nuclear Power Generation/SP-100 Power Generation/	Power Generation/Nominal Power/Sensor Models/Cold Junction Temperature Sensing Model/ Resistance Temperature Detector/Switch ON//OFF Control Block/Switch OFF/MATLAB Function1 ==
  data: u input; y output; Time input
function y = fcn(u,Time)

if Time>=0
    u = NaN;
end

y = u;

== matlab function MCVT_Integration/Sys3 - Power System/Power Generation Subsystem1/Nuclear Power Generation/SP-100 Power Generation/	Power Generation/Nominal Power/Sensor Models/Cold Junction Temperature Sensing Model/ Resistance Temperature Detector/T(Celcuis) vs R ==
  data: R output; T input
function R = fcn(T)

if T >= 0
    R = 100*(1+3.9083*10^(-3)*T-5.775*10^(-7)*T^2);
else
    R = 100*(1+3.9083*10^(-3)*T-5.775*10^(-7)*T^2-4.183*10^(-12)*(T-100)*T^3);
end

== matlab function MCVT_Integration/Sys3 - Power System/Power Generation Subsystem1/Nuclear Power Generation/SP-100 Power Generation/	Power Generation/Nominal Power/Sensor Models/Cold Junction Temperature Sensing Model1/ Resistance Temperature Detector/R vs T ==
  data: R input; T output
function T = fcn(R)

T = 1.76520388802152e-09 * R^4 - 2.98990866289637e-07 * R^3 + 0.000987249006463873 * R^2 ...
    + 2.36178487086887 * R - 245.924688605077;

end

== matlab function MCVT_Integration/Sys3 - Power System/Power Generation Subsystem1/Nuclear Power Generation/SP-100 Power Generation/	Power Generation/Nominal Power/Sensor Models/Cold Junction Temperature Sensing Model1/ Resistance Temperature Detector/RTD with Self-heat Error/MATLAB Function ==
  data: I input; R input; U output
function U = fcn(I, R)

U = I * R;     % Operating current = 1mA
               % Voltage output U = I*R

== matlab function MCVT_Integration/Sys3 - Power System/Power Generation Subsystem1/Nuclear Power Generation/SP-100 Power Generation/	Power Generation/Nominal Power/Sensor Models/Cold Junction Temperature Sensing Model1/ Resistance Temperature Detector/RTD without Self-heat Error/MATLAB Function ==
  data: I input; I output; R input; U output
function [I,U]= fcn(I,R)

U = I * R;     % Operating current = 1mA
               % Voltage output U = I*R

== matlab function MCVT_Integration/Sys3 - Power System/Power Generation Subsystem1/Nuclear Power Generation/SP-100 Power Generation/	Power Generation/Nominal Power/Sensor Models/Cold Junction Temperature Sensing Model1/ Resistance Temperature Detector/Self-heat Calculation/  T vs R  ==
  data: R output; T input
function R = fcn(T)

if T >= 0
    R = 100*(1+3.9083*10^(-3)*T-5.775*10^(-7)*T^2);
else
    R = 100*(1+3.9083*10^(-3)*T-5.775*10^(-7)*T^2-4.183*10^(-12)*(T-100)*T^3);
end

== matlab function MCVT_Integration/Sys3 - Power System/Power Generation Subsystem1/Nuclear Power Generation/SP-100 Power Generation/	Power Generation/Nominal Power/Sensor Models/Cold Junction Temperature Sensing Model1/ Resistance Temperature Detector/Switch ON//OFF Control Block/Switch OFF/MATLAB Function1 ==
  data: u input; y output; Time input
function y = fcn(u,Time)

if Time>=0
    u = NaN;
end

y = u;

== matlab function MCVT_Integration/Sys3 - Power System/Power Generation Subsystem1/Nuclear Power Generation/SP-100 Power Generation/	Power Generation/Nominal Power/Sensor Models/Cold Junction Temperature Sensing Model1/ Resistance Temperature Detector/T(Celcuis) vs R ==
  data: R output; T input
function R = fcn(T)

if T >= 0
    R = 100*(1+3.9083*10^(-3)*T-5.775*10^(-7)*T^2);
else
    R = 100*(1+3.9083*10^(-3)*T-5.775*10^(-7)*T^2-4.183*10^(-12)*(T-100)*T^3);
end

== matlab function MCVT_Integration/Sys3 - Power System/Power Generation Subsystem1/Nuclear Power Generation/SP-100 Power Generation/	Power Generation/Nominal Power/Sensor Models/Cold Junction Temperature Sensing Model2/ Resistance Temperature Detector/R vs T ==
  data: R input; T output
function T = fcn(R)

T = 1.76520388802152e-09 * R^4 - 2.98990866289637e-07 * R^3 + 0.000987249006463873 * R^2 ...
    + 2.36178487086887 * R - 245.924688605077;

end

== matlab function MCVT_Integration/Sys3 - Power System/Power Generation Subsystem1/Nuclear Power Generation/SP-100 Power Generation/	Power Generation/Nominal Power/Sensor Models/Cold Junction Temperature Sensing Model2/ Resistance Temperature Detector/RTD with Self-heat Error/MATLAB Function ==
  data: I input; R input; U output
function U = fcn(I, R)

U = I * R;     % Operating current = 1mA
               % Voltage output U = I*R

== matlab function MCVT_Integration/Sys3 - Power System/Power Generation Subsystem1/Nuclear Power Generation/SP-100 Power Generation/	Power Generation/Nominal Power/Sensor Models/Cold Junction Temperature Sensing Model2/ Resistance Temperature Detector/RTD without Self-heat Error/MATLAB Function ==
  data: I input; I output; R input; U output
function [I,U]= fcn(I,R)

U = I * R;     % Operating current = 1mA
               % Voltage output U = I*R

== matlab function MCVT_Integration/Sys5 - ECLSS/Active Thermal Control System (ATCS)/Faulty Panels/MATLAB Function ==
  data: L_paint_k input; agent input; Time input; dt input; N input; L_paint output; health output; timer_k input; timer output
function [L_paint, health, timer] = paint_fix_with_agent(L_paint_k, agent, Time, dt, N, timer_k)
%% Documentation
% Purpose: Fixing panels with damaged paint
% Date Created: 20 June 2020
% Date Last Modified: 27 July 2021
% Modeler Name: Jaewon Park, CJ Pan (Purdue)
% Funding Acknowledgement: Funded by the NASA RETHi Project (80NSSC19K1076)

% Version Number: MCVT v1.4

% Subsystem Connections: receives input from subsystem 6.

% INPUTS %
%   - Time: time it takes for agents to repair paint damage, unit: hr, type:
%   float
%   - L_paint_k: damaged subsection indices from previous timestep, [1x50],
%   type: integer
%   - agent: agent status, type: integer
%   - dt: time step size, unit: s, type: float
%   - N: total number of panel subsections, type: integer
%   - timer_k: time duration for which agent has been present at previous
%   timestep, s, type: float
    
% OUTPUTS %
%   - L_paint: damaged subsection indices, [1x50], type: integer
%   - health: ratio of damaged subsections to total subsections, type:
%   float
%   - timer: current duration, unit: s, type: float

% Function Dependencies: No dependency

% No Data Depenencies
%% fix damaged paint by agent intervention
time_to_paint = Time*60*60;                            % time to repaint one subsection of a panel
if L_paint_k == 0                                   % if there is no panel to repaint
    L_paint = L_paint_k;                            % output the same result 
    health = 0;                                     % health state is healthy so 0
    timer = 0;                                      % reset timer
else                                                % if there is some panels to repaint
    Damaged_panel = L_paint_k(L_paint_k ~= 0);      % get rid of element with id=0 (These were placeholder values that just maintains the size of stream consistent) 
    n_panel_to_fix = length(Damaged_panel);         % count the number of subsections of the panel to be repainted
    
    if agent == 1                                   % if agent is present
        if timer_k > time_to_paint                  % if elasped time is greater than tiem to paint
            timer = 0;                              % reset timer
            L_paint_k(n_panel_to_fix:end)=0;        % mark panels zero so that you have one less panel to clean
        else                                        % if elasped time is not enough
            timer = timer_k + dt;                   % add time
        end
    else                                            % if there is no agent
       timer = 0;                                   % reset timer
    end
    L_paint = L_paint_k;                            % update the panel data
    health = n_panel_to_fix/N ;                     % update health state, this gives a ratio of panels to be repainted over total number of subsections
end

end

== matlab function MCVT_Integration/Sys3 - Power System/Power Generation Subsystem1/Nuclear Power Generation/SP-100 Power Generation/	Power Generation/Nominal Power/Sensor Models/Cold Junction Temperature Sensing Model2/ Resistance Temperature Detector/Self-heat Calculation/  T vs R  ==
  data: R output; T input
function R = fcn(T)

if T >= 0
    R = 100*(1+3.9083*10^(-3)*T-5.775*10^(-7)*T^2);
else
    R = 100*(1+3.9083*10^(-3)*T-5.775*10^(-7)*T^2-4.183*10^(-12)*(T-100)*T^3);
end

== matlab function MCVT_Integration/Sys3 - Power System/Power Generation Subsystem1/Nuclear Power Generation/SP-100 Power Generation/	Power Generation/Nominal Power/Sensor Models/Cold Junction Temperature Sensing Model2/ Resistance Temperature Detector/Switch ON//OFF Control Block/Switch OFF/MATLAB Function1 ==
  data: u input; y output; Time input
function y = fcn(u,Time)

if Time>=0
    u = NaN;
end

y = u;

== matlab function MCVT_Integration/Sys3 - Power System/Power Generation Subsystem1/Nuclear Power Generation/SP-100 Power Generation/	Power Generation/Nominal Power/Sensor Models/Cold Junction Temperature Sensing Model2/ Resistance Temperature Detector/T(Celcuis) vs R ==
  data: R output; T input
function R = fcn(T)

if T >= 0
    R = 100*(1+3.9083*10^(-3)*T-5.775*10^(-7)*T^2);
else
    R = 100*(1+3.9083*10^(-3)*T-5.775*10^(-7)*T^2-4.183*10^(-12)*(T-100)*T^3);
end
